simulink model "Simulink_Program"  (R2023b)
settings: Solver=FixedStepAuto  StartTime=0.0  StopTime=20  FixedStep=0.001  RelTol=1e-3
PreLoadFcn:
  | % NXP_MBDT_S32K3 callback: DO NOT REMOVE
  | mbd_s32k3.common.nxp.model_callbacks.preLoadFcn();
PostLoadFcn:
  | % NXP_MBDT_S32K3 callback: DO NOT REMOVE
  | mbd_s32k3.common.nxp.model_callbacks.postLoadFcn();
InitFcn:
  | % NXP_MBDT_S32K3 callback: DO NOT REMOVE
  | mbd_s32k3.common.nxp.model_callbacks.initFcn();
undefined symbols (not defined in the file or in its folder): battery_builder_rtmsupport, DiscIntNeverNeededParam, gcbh
libraries: nesl_utility x116, pack_105s34p_structured_with_balancing_lib x105, fl_lib x16, ee_lib x7, simulink x5, BatteryBalancing x1
(part 8 of 8)

== matlab function Simulink_Program/Subsystem/  /contactorControl ==
  settings: updateMethod=DISCRETE
  data: Fault_HVBatRdy input; VCU_DriveReady input; VCU_MainHighVltCntctrCmd input; VCU_DC_CntctrCmd input; Sensors_HVBatteryCurrent_BEV input; Sensors_HVBatteryVoltage_BEV input; Sensors_HVBatteryVoltage_BEV_DCLink input; Sensors_HVBatteryVoltage_BEV_DCFC input; Sensors_HVBatPrecharge_Temp input; Rprecharge input; HVIL_HVBatIntrlk_InternalStat input; K1 output; HVBatPrechgInbt input; K2 output; K3 output; K4 output; HVBatCntctrStat output; Ke_t_HighVoltageBusActualPrechargeTime output; Ke_I_HighVoltageBusShortageCurrent output; Ke_V_HighVoltagePreChargeThreshold output; HVBatPrechgInbt output
function [K1, K2, K3, K4, HVBatCntctrStat, Ke_t_HighVoltageBusActualPrechargeTime, Ke_I_HighVoltageBusShortageCurrent, Ke_V_HighVoltagePreChargeThreshold, HVBatPrechgInbt] = fcn(Fault_HVBatRdy, VCU_DriveReady, VCU_MainHighVltCntctrCmd, VCU_DC_CntctrCmd, Sensors_HVBatteryCurrent_BEV, Sensors_HVBatteryVoltage_BEV, Sensors_HVBatteryVoltage_BEV_DCLink, Sensors_HVBatteryVoltage_BEV_DCFC, Sensors_HVBatPrecharge_Temp, Rprecharge, HVIL_HVBatIntrlk_InternalStat, HVBatPrechgInbt)
%-----------------------------------------------------------
% McMaster Automotive Resource Centre (MARC)
% Electrical Engineer: Romulo Vieira, M.Sc and MBA - PhD Candidate
%-----------------------------------------------------------
% Purpose: Manage precharge relay and contactor sequence with retry + inhibit logic
%-----------------------------------------------------------
% HVBatCntctrStat Mapping:
% 0 – Idle/Waiting
% 1 – Precharge Active
% 2 – Precharge Complete (Main contactors closed)
% 3 – Precharge Failed (necessary cooldown after unique failures of voltage and current)
% 4 – Inhibit State (necessary cooldown after multiple failures)
% 5 - DCFC Charging
% 6 – Contactor Control Shorted High
% 7 - Contactor Control Shorted Low
% VCU_MainHighVltCntctrCmd mapping:
% 0 - open
% 1 - closed
% 2 - impact_open
% 3 - fast_open
% 7 - invalid
% --- Constants ---
Unset_VbusTargetHitIdx             = -1;   % Flag indicating Vbus threshold not yet hit
Min_Samples                        = 0;    % Minimum number of samples where relays and contactor are both active
% Internal Configuration Parameters
Ke_t_PrechargeFailCoolTime             = 1000;   % Cooldown time after one single failed attempt [ms]
Ke_t_ExtraPrechargeFailCoolPenaltyTime = 1000;   % Additional cooldown after two multiple failures [ms]
Ke_t_PrechargeFailMaxPenalty           = 10000;  % Maximum time threshold before enabling precharge inhibit state
Ke_t_BPCM_Precharge_Fail_Penalty_Time  = 15000;  % Hard inhibit time if failures persist [ms]
Ke_I_HighVoltageBusShortageCurrentMin  = 10.8;   % Minimum expected precharge current [A] (Pack current ≥ 0.6 * (pack voltage / Precharge resistance))
                                                 % // max pack voltage = 450 V, Precharge resistance = 25 ohm
Ke_V_HighVoltagePreChargeThreshold     = 0.98 * Sensors_HVBatteryVoltage_BEV;  % Target voltage threshold
Ke_t_HighVoltageBusPrechargeTime       = 100;    % Maximum allowable time for precharging [ms]
% --- Persistent Variables ---
persistent I_buffer idx HVBatCntctrStat_Aux VbusTargetHitIdx ...
    t_counter last_PrechargeAllw Prchrgpnltytimer PrechargeFailCount last_HVBatCntctrStat_Aux
if isempty(I_buffer)
    I_buffer               = zeros(Ke_t_HighVoltageBusPrechargeTime,1);
    idx                    = 1;
    HVBatCntctrStat_Aux    = 0;
    VbusTargetHitIdx       = Unset_VbusTargetHitIdx;
    t_counter              = 0;
    last_PrechargeAllw     = 0;
    Prchrgpnltytimer       = 0;
    PrechargeFailCount     = 0; % Represented by k_fail in the flowchart
    last_HVBatCntctrStat_Aux = 0;
end
% --- Default Outputs ---
K1 = 0; K2 = 0; K3 = 0; K4 = 0;
HVBatCntctrStat = HVBatCntctrStat_Aux;
Ke_t_HighVoltageBusActualPrechargeTime = 0;
Ke_I_HighVoltageBusShortageCurrent = 0;
if VCU_MainHighVltCntctrCmd == 3 % VCU_MainHighVltCntctrCmd = 3 means FAST_OPEN
    HVBatCntctrStat_Aux = 0;
end
% --- State Machine ---
switch HVBatCntctrStat_Aux
    case 0 % OPEN STATE
        % Set the Battery Contactor States
        K1 = 0; K2 = 0; K3 = 0; K4 = 0;
        % Check Initial conditions
        % HVIL_HVBatIntrlk_InternalStat = 1 (pass),
        % HVIL_HVBatIntrlk_InternalStat = 2 (fail)
        % For precharge initiation HVIL_HVBatIntrlk_InternalStat must pass.
        if VCU_MainHighVltCntctrCmd == 1 && HVBatPrechgInbt == 0 && HVIL_HVBatIntrlk_InternalStat == 1 && Fault_HVBatRdy == 1 && VCU_DriveReady == 1 && Sensors_HVBatPrecharge_Temp < 60
            I_buffer(:) = 0;
            idx = 1;
            VbusTargetHitIdx = Unset_VbusTargetHitIdx;
            t_counter = 0;
            HVBatCntctrStat_Aux = 1;
        end

        %% Shorted High
        
        if VCU_MainHighVltCntctrCmd == 0 && Sensors_HVBatteryVoltage_BEV_DCLink > 270 
            HVBatCntctrStat_Aux = 6;
        end

    case 1  % PRECHARGING STATE
        % Abort if initial conditions are no longer valid
        if VCU_MainHighVltCntctrCmd == 0 || HVBatPrechgInbt == 1 || HVIL_HVBatIntrlk_InternalStat == 2 || Fault_HVBatRdy == 0 || VCU_DriveReady == 0 || Sensors_HVBatPrecharge_Temp >= 60
            HVBatCntctrStat_Aux = 0;
        end
        % Set precharge relays ON
        K1 = 0; K2 = 1; K3 = 1; K4 = 0;
        %
        % if Fault_K1_Shorted_Low_Condition == 1
        %     HVBatCntctrStat_Aux = 7; % K1 Shorted Low
        % end
        % if Fault_K4_Shorted_Low_Condition == 1
        %     HVBatCntctrStat_Aux = 7; % K4 Shorted Low
        % end
        % Record current
        if t_counter == 0
            I_buffer(idx) = Sensors_HVBatteryVoltage_BEV / Rprecharge;
        else
            I_buffer(idx) = Sensors_HVBatteryCurrent_BEV;
        end
        Ke_I_HighVoltageBusShortageCurrent = max(I_buffer);
        % Voltage target check
        if VbusTargetHitIdx == Unset_VbusTargetHitIdx && Sensors_HVBatteryVoltage_BEV_DCLink >= Ke_V_HighVoltagePreChargeThreshold
            VbusTargetHitIdx = t_counter + 1;
        end
        % Time since start or since target hit
        if VbusTargetHitIdx ~= Unset_VbusTargetHitIdx
            delta_samples = VbusTargetHitIdx;
        else
            delta_samples = t_counter;
        end
        % SUCCESS - PRECHARGE CONDITIONS ARE MET
        if t_counter < Ke_t_HighVoltageBusPrechargeTime && ...
                VbusTargetHitIdx ~= Unset_VbusTargetHitIdx && delta_samples < Ke_t_HighVoltageBusPrechargeTime && Ke_I_HighVoltageBusShortageCurrent >= Ke_I_HighVoltageBusShortageCurrentMin
            
            % Set the next Battery Contactor Status
            HVBatCntctrStat_Aux = 2;
            % Set the Battery Contactor States
            K1 = 1; K2 = 0; K3 = 0; K4 = 0;
            PrechargeFailCount = 0;
            Prchrgpnltytimer = 0;
        % FAILURE - PRECHARGE CONDITIONS ARE NOT MET
        elseif t_counter == Ke_t_HighVoltageBusPrechargeTime && ...
               (VbusTargetHitIdx == Unset_VbusTargetHitIdx || delta_samples == Ke_t_HighVoltageBusPrechargeTime || Ke_I_HighVoltageBusShortageCurrent < Ke_I_HighVoltageBusShortageCurrentMin)
            % Set the Battery Contactor States
            K1 = 0; K2 = 0; K3 = 0; K4 = 0;
           % Set the Battery Contactor Status
            HVBatCntctrStat_Aux = 3;
            % Update the total cooling time before re-attempt
            PrechargeFailCount = PrechargeFailCount + 1;
            Prchrgpnltytimer = Prchrgpnltytimer + Ke_t_PrechargeFailCoolTime;
            if PrechargeFailCount >= 2
                Prchrgpnltytimer = Ke_t_ExtraPrechargeFailCoolPenaltyTime;
            end
            if PrechargeFailCount >= 10
                HVBatCntctrStat_Aux = 4;
                HVBatPrechgInbt = 1;
                Prchrgpnltytimer = Ke_t_BPCM_Precharge_Fail_Penalty_Time;
            end
        end
        % Update counters
        t_counter = t_counter + 1;
        idx = idx + 1;
        if idx > Ke_t_HighVoltageBusPrechargeTime, idx = 1; end
    case 2  % PRECHARGE COMPLETE OR CLOSED
        % Set the Battery Contactor States
        K1 = 1; K2 = 0; K3 = 0; K4 = 0;
        % If statement to detect DCFC charging
        if VCU_DC_CntctrCmd == 1
            HVBatCntctrStat_Aux = 5; % DCFC CHARGING
        end
        if VCU_MainHighVltCntctrCmd == 0
            HVBatCntctrStat_Aux = 0;
        end

        if VCU_MainHighVltCntctrCmd == 1 && Sensors_HVBatteryVoltage_BEV_DCLink < 270
            HVBatCntctrStat_Aux = 7;
        end

    case 3 % FAULT: PRECHARGE FAILED STATE 4 --> 3
        % Set the Battery Contactor States
        K1 = 0; K2 = 0; K3 = 0; K4 = 0;
        if Prchrgpnltytimer > 0
            Prchrgpnltytimer = Prchrgpnltytimer - 1;
        else
            HVBatCntctrStat_Aux = 0;
        end
    case 4  % FAULT: PRECHARGE INHIBIT STATE - 5 -- > 4
        % Set the Battery Contactor States
        K1 = 0; K2 = 0; K3 = 0; K4 = 0;
        if Prchrgpnltytimer > 0
            Prchrgpnltytimer = Prchrgpnltytimer - 1;
        else
        Prchrgpnltytimer = 0;
        HVBatPrechgInbt = 0;
        HVBatCntctrStat_Aux = 0;
        PrechargeFailCount = 0;
        end
    % Added by Tridib: New DCFC case -3 -- > 5
    case 5 % DCFC CHARGING
        % Set the Battery Contactor States
        K1 = 1; K2 = 0; K3 = 0; K4 = 1;
        if VCU_DC_CntctrCmd == 0
            if VCU_MainHighVltCntctrCmd == 0
                HVBatCntctrStat_Aux = 0; % switch to OPEN
            else
                HVBatCntctrStat_Aux = 2; % switch to CLOSED
            end
        end
    
    case 6 % FAULT: Shorted High
       
        if VCU_MainHighVltCntctrCmd == 0 && Sensors_HVBatteryVoltage_BEV_DCLink < 270
            HVBatCntctrStat_Aux = 0;
        end

    case 7 % FAULT: Shorted Low

        if VCU_DC_CntctrCmd == 0 && Sensors_HVBatteryVoltage_BEV_DCFC < 270 && VCU_MainHighVltCntctrCmd == 1 && Sensors_HVBatteryVoltage_BEV_DCLink > 270
            HVBatCntctrStat_Aux = 2;
        end
    otherwise
        K1 = 0; K2 = 0; K3 = 0; K4 = 0;
        HVBatCntctrStat_Aux = 0;
end
% --- Final Outputs ---
HVBatCntctrStat = HVBatCntctrStat_Aux;
Ke_t_HighVoltageBusActualPrechargeTime = max(0, VbusTargetHitIdx + ...
    Min_Samples * (VbusTargetHitIdx ~= Unset_VbusTargetHitIdx));
last_PrechargeAllw = double(Fault_HVBatRdy);
last_HVBatCntctrStat_Aux = HVBatCntctrStat_Aux;
end

== matlab function Simulink_Program/Subsystem/Cell Balancing/MATLAB Function ==
  data: HVBatCell_Voltages input; BalCmd output; Enable input; isBalancingActive output

function [BalCmd, isBalancingActive] = PassiveBalanceController(HVBatCell_Voltages, Enable)
% PassiveBalanceController - Controls passive cell balancing and detects faults
% Inputs:
%   HVBatCell_Voltages - Vector of cell voltages
%   Enable - Boolean flag to enable balancing
% Outputs:
%   BalCmd - Logical vector of balancing commands (true = balance)
%   isBalancingActive - Logical flag indicating if any balancing is active

%#codegen

% Parameters
V_thresh = 0.05; % Threshold in Volts (50 mV)
N = length(HVBatCell_Voltages);
BalCmd = false(N, 1);

% Check if balancing is enabled
if Enable
    % Find minimum cell voltage
    V_min = min(HVBatCell_Voltages);

    % Loop through each cell
    for i = 1:N
        deltaV = HVBatCell_Voltages(i) - V_min;

        % Normal balancing condition
        if deltaV > V_thresh
            BalCmd(i) = true;
        else
            BalCmd(i) = false;
        end

        % Fault detection (optional logging)
        if deltaV <= V_thresh && BalCmd(i)
            fprintf('Stuck Closed Fault detected on Cell %d\n', i);
        elseif deltaV > V_thresh && ~BalCmd(i)
            fprintf('Stuck Open Fault detected on Cell %d\n', i);
        end
    end
end

% Balancing active flag: at least one command is true
isBalancingActive = any(BalCmd);

end

== matlab function Simulink_Program/Subsystem/Plug-In Charging/ac_plugin_charging ==
  settings: updateMethod=DISCRETE
  data: HVBat_DC_CntctrStat output; Time input; DCChargeInitialized input; MainHighVltCntctrCmd input; ChargingSysSts input; Charger_Plugin_Status input; HVBatCell_Voltage_High_Thrsh input; Fault_General input; HVBatModTempMax input; HVBatCellVltMax input; HVBatSOCMax input; K1 input; K2 input; K3 input; HVBatChargeStat output; MaxChargeCurrentAllowed output; MaxChargeRegenPowerAllowed output; HVBatMaxCellVltAlld output; HVBatMaxPkVltAllwd output
function [HVBat_DC_CntctrStat, HVBatChargeStat, MaxChargeCurrentAllowed, MaxChargeRegenPowerAllowed, HVBatMaxCellVltAlld, HVBatMaxPkVltAllwd] = ac( ...
    Time, DCChargeInitialized, MainHighVltCntctrCmd, ChargingSysSts, Charger_Plugin_Status, ...
    HVBatCell_Voltage_High_Thrsh, Fault_General, ...
    HVBatModTempMax, HVBatCellVltMax, HVBatSOCMax, K1, K2, K3)

% ===========================
%% INPUTS
% Time - Current time (s)
% DC_CntctrCmd - Command from VCU to close DCFC contactor (0/1/2/3/7)
% MainHighVltCntctrCmd - VCU command for main contactor (0/1/2/3/7)
% K4,K3,K2,K1 - Physical contactor feedbacks (1=closed, 0=open)
% Fault_General - Fault flag (0=OK, 1=Fault/Weld)
% ChargingSysSts - 0=Not Charging,1=Charging,2=Interrupted,3=Complete
% Charger_Plugin_Status - 0=Unplugged, 1=Plugged
% HVBatCell_Voltage_High_Thrsh - Max allowable cell voltage (V)
% HVBatModTempMax, HVBatCellVltMax, HVBatSOCMax - vectors
% VDC - DC bus voltage (V)
% HVBatMaxPkVltAllwd 
% HVBatMaxCellVltAlld

%% OUTPUTS
% HVBat_DC_CntctrStat - 0=Open, 1=Closed, 2=Faulted
% HVBatChargeStat     - 0=Not Ready, 1=Ready, 2=Charging, 3=Fault, 6=Complete
% MaxChargeCurrentAllowed - Allowed charging current (A)
% P_charge            - Estimated DC charging power (W)

%% --- Lookup Table for Max Charge Current [SOC vs Temp] ---
SOC = [0 0.05 0.10 0.15 0.20 0.25 0.30 0.35 0.40 0.45 0.50 0.55 0.60 0.65 0.70 0.75 0.80 0.85 0.90 0.95 1.00]; % 19 + 2 points
Temp = [-25 -20 -10 0 5 10 20 25 35 40 50]; % 11 points

ACChgCurrentProfile = [ ...
  0.05 0.05 0.05 0.05 0.05 0.05 0.05 0.05 0.05 0.05 0.05 0.05 0.05 0.02 0.02 0.01 0.01 0.01 0.01 0.01 0.00; ...
  0.06 0.06 0.06 0.06 0.06 0.06 0.06 0.06 0.06 0.06 0.06 0.06 0.06 0.02 0.02 0.02 0.02 0.01 0.01 0.01 0.00; ...
  0.45 0.45 0.45 0.45 0.45 0.15 0.15 0.15 0.15 0.09 0.09 0.09 0.09 0.03 0.03 0.03 0.03 0.02 0.02 0.02 0.00; ...
  0.70 0.70 0.70 0.70 0.70 0.70 0.70 0.70 0.70 0.50 0.50 0.50 0.50 0.25 0.25 0.25 0.25 0.10 0.10 0.09 0.00; ...
  0.85 0.85 0.85 0.85 0.85 0.85 0.85 0.85 0.85 0.75 0.75 0.75 0.75 0.63 0.63 0.58 0.44 0.35 0.24 0.12 0.00; ...
  0.88 0.88 0.88 0.88 0.88 0.88 0.88 0.88 0.88 0.88 0.88 0.88 0.88 0.88 0.88 0.68 0.59 0.35 0.24 0.12 0.00; ...
  0.88 0.88 0.88 0.88 0.88 0.88 0.88 0.88 0.88 0.88 0.88 0.88 0.88 0.88 0.88 0.88 0.74 0.59 0.44 0.29 0.00; ...
  0.88 0.88 0.88 0.88 0.88 0.88 0.88 0.88 0.88 0.88 0.88 0.88 0.88 0.88 0.88 0.88 0.88 0.74 0.59 0.44 0.00; ...
  0.88 0.88 0.88 0.88 0.88 0.88 0.88 0.88 0.88 0.88 0.88 0.88 0.88 0.88 0.88 0.88 0.88 0.74 0.65 0.59 0.00; ...
  0.88 0.88 0.88 0.88 0.88 0.88 0.88 0.88 0.88 0.88 0.88 0.88 0.88 0.88 0.88 0.88 0.88 0.76 0.74 0.60 0.00; ...
  0.88 0.88 0.88 0.88 0.88 0.88 0.88 0.88 0.88 0.88 0.88 0.88 0.88 0.88 0.88 0.88 0.88 0.60 0.60 0.48 0.00 ...
];

%% --- Calibrations ---
persistent Ke_t_DCFC_CntctrOpn Ke_T_HighChrgTempThresholdIn Ke_T_LowChrgTempThresholdIn ...
           Ke_T_HighChrgTempThresholdOut Ke_T_LowChrgTempThresholdOut Ke_U_ChargeCompletionVlt ...
           ParallelCellNum Ke_t_ChargeCompletionHold HVBatChargeTimer ...
           chargeCompleteFlag overTempFlag
if isempty(Ke_t_DCFC_CntctrOpn)
    Ke_t_DCFC_CntctrOpn = 1.5;           
    Ke_T_HighChrgTempThresholdIn = 50;   
    Ke_T_LowChrgTempThresholdIn  = -25;  
    Ke_T_HighChrgTempThresholdOut = 48;  
    Ke_T_LowChrgTempThresholdOut  = -23; 
    Ke_U_ChargeCompletionVlt = 4.14;     
    ParallelCellNum = 34;
    Ke_t_ChargeCompletionHold = 0.3;     % seconds
    HVBatChargeTimer = 0;
    chargeCompleteFlag = false;
    overTempFlag = false;
end


persistent Ke_RegenVltMargin Ke_P_MaxChargeRegen
if isempty(Ke_RegenVltMargin)
    Ke_RegenVltMargin   = 0.05;   % [V] margin below HVBatCell_Voltage_High_Thrsh
    Ke_P_MaxChargeRegen = 11000;  % [W] max allowed regen during charge
end

%% --- Persistent states ---
persistent HVBatChargeStat_Aux DC_FaultDetectedTime DC_WeldDetected MaxChargeCurrentAllowed_Aux MaxChargeRegenPowerAllowed_Aux
if isempty(HVBatChargeStat_Aux)
    HVBatChargeStat_Aux = 0;
    DC_FaultDetectedTime = -1;
    DC_WeldDetected = false;
    MaxChargeCurrentAllowed_Aux = 0;
    MaxChargeRegenPowerAllowed_Aux = 0;
end

%% Default outputs
HVBatChargeStat = HVBatChargeStat_Aux;
MaxChargeCurrentAllowed = MaxChargeCurrentAllowed_Aux;
MaxChargeRegenPowerAllowed = MaxChargeRegenPowerAllowed_Aux;

%% Plug-in readiness
if (Charger_Plugin_Status == 1) && (DCChargeInitialized == 0) && ~chargeCompleteFlag && overTempFlag == false
    HVBatChargeStat_Aux = 0; % Not Ready
    % Battery is able to accept charge
    if (MainHighVltCntctrCmd == 1) && (K1 == 1) && (K2 == 0) && (K3 == 0) && ~chargeCompleteFlag && overTempFlag == false && Fault_General == 0
        HVBatChargeStat_Aux = 1;   % Ready
    elseif (MainHighVltCntctrCmd == 1) && (K1 == 1) && (K2 == 0) && (K3 == 0) && ~chargeCompleteFlag && overTempFlag == false && Fault_General == 1
        HVBatChargeStat_Aux = 0;   % Not Ready
    end
elseif Charger_Plugin_Status == 1 && DCChargeInitialized == 0 && chargeCompleteFlag && overTempFlag == false
        MaxChargeCurrentAllowed_Aux = 0;
        HVBatChargeStat_Aux = 6;       % Complete
else
    HVBatChargeStat_Aux = 0;
    MaxChargeCurrentAllowed_Aux = 0;
    MaxChargeRegenPowerAllowed_Aux = 0;
    DC_FaultDetectedTime = -1;
    DC_WeldDetected = false;
end

%% Charging logic
handshake_ok = (HVBatChargeStat_Aux == 1 && ChargingSysSts == 1);
if handshake_ok
    HVBatChargeStat_Aux = 2; % charge allow current
end

Vmax = max(HVBatCellVltMax);
Temp_actual = max(HVBatModTempMax);
SOC_actual  = max(HVBatSOCMax);

if overTempFlag == true
    if Temp_actual <= (Ke_T_HighChrgTempThresholdIn - 5) && Temp_actual >= (Ke_T_LowChrgTempThresholdIn + 5)
        overTempFlag = false;
    end
end

charge_allow_threshold = Ke_U_ChargeCompletionVlt;

if HVBatChargeStat_Aux == 2 && (ChargingSysSts == 1) && (Vmax < charge_allow_threshold) && chargeCompleteFlag == false && overTempFlag == false && Temp_actual < Ke_T_HighChrgTempThresholdIn && Temp_actual > Ke_T_LowChrgTempThresholdIn
    MaxChargeCurrentAllowed = ParallelCellNum * interp2(SOC, Temp, ACChgCurrentProfile, SOC_actual, Temp_actual);
      
    MaxChargeCurrentAllowed_Aux = MaxChargeCurrentAllowed;
    HVBatChargeStat_Aux = 2; % charge allow current
    
% Charge completion    
elseif (ChargingSysSts == 1) && (Vmax >= (HVBatCell_Voltage_High_Thrsh - 0.01))
        % Enter CV-hold mode: provide PackCVCurrent to hold voltage steady
        MaxChargeCurrentAllowed_Aux = 0;
        HVBatChargeStat_Aux = 6;       % Complete
        chargeCompleteFlag = true;

elseif Temp_actual >= Ke_T_HighChrgTempThresholdIn || Temp_actual <= Ke_T_LowChrgTempThresholdIn
    % Not in charging handshake/phase: ensure DCFC current 0
    MaxChargeCurrentAllowed_Aux = 0;
    overTempFlag = true;
    HVBatChargeStat_Aux = 3;
end

if ChargingSysSts == 1
    %% Regen power limit during charge mode
     Vcell_max = max(HVBatCellVltMax);

    if Vcell_max >= HVBatCell_Voltage_High_Thrsh
        % No regen allowed at or above max allowable cell voltage
        MaxChargeRegenPowerAllowed = 0;
        MaxChargeRegenPowerAllowed_Aux = MaxChargeRegenPowerAllowed;

    elseif Vcell_max >= (HVBatCell_Voltage_High_Thrsh - Ke_RegenVltMargin)
        % Linearly reduce regen as voltage approaches limit
        scale = (HVBatCell_Voltage_High_Thrsh - Vcell_max) / Ke_RegenVltMargin;
        MaxChargeRegenPowerAllowed = max(0, scale * Ke_P_MaxChargeRegen);
        MaxChargeRegenPowerAllowed_Aux = MaxChargeRegenPowerAllowed;

    else
        % Full regen allowed (charge-mode limited)
        MaxChargeRegenPowerAllowed = Ke_P_MaxChargeRegen;
        MaxChargeRegenPowerAllowed_Aux = MaxChargeRegenPowerAllowed;
    end
end

% Fault handling
if ChargingSysSts == 1 && Fault_General == 1 
    HVBatChargeStat_Aux = 3;
    %P_charge = 0;
else
    DC_FaultDetectedTime = -1;
end

%% Charge completion
if max(HVBatCellVltMax) >= Ke_U_ChargeCompletionVlt
    % if HVBatChargeTimer < 0
    %     HVBatChargeTimer = Time; % latch the first detection time
    % end
    % 
    % % Check if hold duration has passed
    % if (Time - HVBatChargeTimer) >= Ke_t_ChargeCompletionHold
        MaxChargeCurrentAllowed_Aux = 0;
        HVBatChargeStat_Aux = 6;
        chargeCompleteFlag = true;
        MaxChargeRegenPowerAllowed_Aux = 0;
    % end
else
    HVBatChargeTimer = -1; % reset latch when voltage drops below threshold
    chargeCompleteFlag = false;
end

if (ChargingSysSts == 3)
    MaxChargeCurrentAllowed_Aux = 0;
    HVBatChargeStat_Aux = 6;       % Complete
    chargeCompleteFlag = true;
end

%% Overvoltage handling
if max(HVBatCellVltMax) > HVBatCell_Voltage_High_Thrsh
    HVBatChargeStat_Aux = 3;
    % P_charge = 0;
end

%% Outputs
HVBatChargeStat = HVBatChargeStat_Aux;
MaxChargeCurrentAllowed = MaxChargeCurrentAllowed_Aux;
MaxChargeRegenPowerAllowed = MaxChargeRegenPowerAllowed_Aux;
if isnan(MaxChargeCurrentAllowed), MaxChargeCurrentAllowed = 0; end
if isnan(MaxChargeRegenPowerAllowed), MaxChargeRegenPowerAllowed = 0; end

%% --- Step 3: Update Max Voltages
HVBatMaxCellVltAlld = HVBatCell_Voltage_High_Thrsh;
HVBatMaxPkVltAllwd = HVBatCell_Voltage_High_Thrsh * 105; % example for 105 series cells

HVBat_DC_CntctrStat = 0;
end

== matlab function Simulink_Program/Subsystem/Plug-In Charging/MATLAB Function ==
  data: DCChargeInitialized input; MaxChargeCurrentAllowed_AC input; output output; MaxChargeCurrentAllowed_DC input
function output = fcn(DCChargeInitialized, MaxChargeCurrentAllowed_AC, MaxChargeCurrentAllowed_DC)
    if DCChargeInitialized == 0
        output = MaxChargeCurrentAllowed_AC;
    else
        output = MaxChargeCurrentAllowed_DC;
    end

== matlab function Simulink_Program/Subsystem/Plug-In Charging/dcfc ==
  settings: updateMethod=DISCRETE
  data: DC_Isolation_Sts output; HVBat_DC_CntctrStat output; HVBatChargeStat output; Time input; DCChargeInitialized input; MaxChargeCurrentAllowed output; PlugInChargingCmd input; DC_Isolation_Cmd input; MainHVBatCntctrCmd input; ChargingSysSts input; Charger_Plugin_Status input; HVBatCell_Voltage_High_Thrsh input; Fault_General input; temperatureCell input; voltageCell input; socCell input; K1 input; K2 input; K3 input; HVBat_DC_CntctrOpn output; HVBatCntctrOpn output; HVBatCntctrReq output; K4 input
function [DC_Isolation_Sts, HVBat_DC_CntctrStat, HVBatChargeStat, MaxChargeCurrentAllowed, HVBat_DC_CntctrOpn, HVBatCntctrOpn, HVBatCntctrReq] = dcfc(Time, DCChargeInitialized, PlugInChargingCmd, DC_Isolation_Cmd, MainHVBatCntctrCmd, ChargingSysSts, Charger_Plugin_Status, HVBatCell_Voltage_High_Thrsh, Fault_General, temperatureCell, voltageCell, socCell, K1, K2, K3, K4)

% ===========================
%% INPUTS
% Time - current absolute time (s)
% PlugInChargingCmd - Command from VCU indicating vehicle is plugged into DCFC station and ready to close DCFC contactor (K4) (0 - Open, 1 - Closed, 2 - Impact Open, 3 - Fast Open, 7 - SNA).
% MainHVBatCntctrCmd - Command from VCU indicating main contactor (K1) is commanded to close (0 - Open, 1 - Closed, 2 - Impact Open, 3 - Fast Open, 7 - SNA).
% K4 - Physical feedback (status of DCFC contactor) (1 - physically closed, 0 - physically open).
% K3 - Physical feedback (status of precharge (+) contactor) (1 - physically closed, 0 - physically open).
% K2 - Physical feedback (status of precharge (-) contactor) (1 - physically closed, 0 - physically open).
% K1 - Physical feedback (status of main contactor) (1 - physically closed, 0 - physically open).
% Fault_General - Indicate if DCFC contactor driver circuit fault or a weld is detected (0 - normal, 1 - at least 1 fault detected).
% DC_Isolation_Cmd - Command from VCU to disable isolation monitoring when DCFC active (0 - Enable, 1 - Disable).
% ChargingSysSts - Command from VCU and indicates the status of the charging function (0 - Not Charging, 1 - Charging, 2 - ChargeInterrupted, 3 - ChargeComplete, 7 - SNA). 
% Charger_Plugin_Status - Signal from charge port. True if DCFC connector is plugged in (0 - Charger_Unplugged, 1 - Charger_Plugged_In).
% HVBatPack_Voltage - Current HV pack voltage (V).
% MaxCellVoltage_V - Highest measured cell voltage (V).
% HVBatCell_Voltage_High_Thrsh - Signal from the BPCM indicating the maximum cell voltage that the battery pack needs to be below in order to become functional. Above this value, the BPCM will set HVBatCntctrReq to 1, and HVBatCntctrOpn to 1 after appropriate maturation time. If above this value during power up, HVBatRdy will also be set to 0.
% cellTemperature - All cell temperatures in degC.
% cellVoltage - All cell voltages in V.
% cellSOC - All cell SOCs in percentages.
% ===========================
%% OUTPUTS
% HVBat_DC_CntctrStat - DCFC contactor status (0 - Open, 1 - Closed, 2 - Faulted).
% HVBatChargeStat - Sent out by the BPCM as an indication of its present status (0 - Not Ready, 1 - Ready, 2 - Complete).
% DC_Isolation_Sts - Isolation status for DC charging (0 - Enable, 1 - Disable).
% HVBatMaxCellVltAlld - Max allowable cell voltage (V).
% HVBatMaxPkVltAllwd - Max allowable pack voltage (V).
% MaxChargeCurrentAllowed - Max allowable DCFC current (A).
% HVBatCntctrReq - Request to open contactors (fault/weld) (0 - No request to open, 1 - Request to open).
% HVBatCntctrOpn - Command to DCFC contactor coil (Not Commanded Open, 1 - Commanded Open).
%% CALIBRATIONS (Constants/Tunable Parameters)
% MaxChargeCurrentDefault - Max allowable DCFC charging current (Default = 30 A).
% Ke_T_HighChrgTempThresholdIn - Upper limit for enabling charging (Default = 50 deg C).
% Ke_T_HighChrgTempThresholdOut - Upper hysteresis threshold (Default = 48 deg C).
% Ke_T_LowChrgTempThresholdIn - Lower limit for enabling charging (Default = -25 deg C).
% Ke_T_LowChrgTempThresholdOut - Lower hysteresis threshold (Default = -23 deg C).
% Ke_U_ChargeCompletionVlt - Voltage threshold for charge completion (Default = 4.14 V).
% Ke_t_DCFC_CntctrOpn - Delay before opening contactor after fault (Default = 1.5 sec).
% ParallelCellNum - Number of parallel cell, which dictates pack current (34).
%% --- Lookup Table for AC Max Charge Current [SOC vs Temp]
SOC = [0 0.05 0.10 0.15 0.20 0.25 0.30 0.35 0.40 0.45 0.50 0.55 0.60 0.65 0.70 0.75 0.80 0.85 0.90 0.95 1.00]; % 19 + 2 points
Temp = [-25 -20 -10 0 5 10 20 25 35 40 50]; % 11 points
DCChgCurrentProfile = [ ...
  0.05 0.05 0.05 0.05 0.05 0.05 0.05 0.05 0.05 0.05 0.05 0.05 0.05 0.02 0.02 0.01 0.01 0.01 0.01 0.01 0.00; ...
  0.06 0.06 0.06 0.06 0.06 0.06 0.06 0.06 0.06 0.06 0.06 0.06 0.06 0.02 0.02 0.02 0.02 0.01 0.01 0.01 0.00; ...
  0.45 0.45 0.45 0.45 0.45 0.15 0.15 0.15 0.15 0.09 0.09 0.09 0.09 0.03 0.03 0.03 0.03 0.02 0.02 0.02 0.00; ...
  0.70 0.70 0.70 0.70 0.70 0.70 0.70 0.70 0.70 0.50 0.50 0.50 0.50 0.25 0.25 0.25 0.25 0.10 0.10 0.10 0.00; ...
  0.85 0.85 0.85 0.85 0.85 0.85 0.85 0.85 0.85 0.75 0.75 0.75 0.75 0.63 0.63 0.58 0.58 0.35 0.35 0.20 0.00; ...
  1.00 1.00 1.00 1.00 1.00 1.00 1.00 1.00 1.00 1.00 1.00 1.00 1.00 1.00 1.00 0.90 0.90 0.60 0.60 0.30 0.00; ...
  1.33 1.33 1.33 1.33 1.33 1.33 1.33 1.33 1.33 1.33 1.33 1.33 1.33 1.33 1.33 1.30 1.30 0.80 0.80 0.50 0.00; ...
  1.50 1.50 1.50 1.50 1.50 1.50 1.50 1.50 1.50 1.50 1.50 1.50 1.50 1.50 1.50 1.50 1.50 0.90 0.90 0.60 0.00; ...
  2.17 2.17 2.17 2.17 2.17 2.17 2.17 2.17 2.17 2.17 2.17 2.17 2.17 1.83 1.83 1.50 1.50 0.90 0.90 0.60 0.00; ...
  2.50 2.50 2.50 2.50 2.50 2.50 2.50 2.50 2.50 2.50 2.50 2.50 2.50 2.00 2.00 1.50 1.50 0.90 0.90 0.60 0.00; ...
  1.50 1.50 1.50 1.50 1.50 1.50 1.50 1.50 1.50 1.50 1.50 1.50 1.50 1.50 1.50 1.00 1.00 0.60 0.60 0.48 0.00  ...
];
%% --- Calibration (tunable) ---
% --- Contactor timing calibrations ---
persistent MaxChargeCurrentDefault ...
           Ke_T_HighChrgTempThresholdIn Ke_T_LowChrgTempThresholdIn ...
           Ke_T_HighChrgTempThresholdOut Ke_T_LowChrgTempThresholdOut ...
           Ke_U_ChargeCompletionVlt Ke_U_ChargeCompletionHyst ...
           ParallelCellNum Ke_t_ChargeCompletionHold  ...
           Ke_CV_PerCell_A Ke_MinCV_PackCurrent Ke_DisableBalDuringCV ...
           chargeCompleteFlag overTempFlag ...
           Ke_t_DCFC_CntctrOpn Ke_t_CntctrReqDelay
if isempty(MaxChargeCurrentDefault)
    % Ke_t_DCFC_CntctrOpn = 1.5;           % seconds (1500 ms) - time between HVBatCntctrOpn and actual coil open
    % Ke_t_CntctrReqDelay = 30.0;          % seconds - time HVBatCntctrReq must persist before notifying HVBatCntctrOpn
    Ke_t_DCFC_CntctrOpn = 0;
    Ke_t_CntctrReqDelay = 0;  
    MaxChargeCurrentDefault = 30;        % A (plugin default)
    Ke_T_HighChrgTempThresholdIn = 50;   % degC
    Ke_T_LowChrgTempThresholdIn  = -25;  % degC
    Ke_T_HighChrgTempThresholdOut = 48;  % degC
    Ke_T_LowChrgTempThresholdOut  = -23; % degC
    Ke_U_ChargeCompletionVlt = 4.19;     % V per cell held for completion (example)
    Ke_U_ChargeCompletionHyst = 0.005;   % 5 mV hysteresis (tunable)
    ParallelCellNum = 34; 
    Ke_t_ChargeCompletionHold = 0.3;     % seconds
    % CV-hold calibration (tunable)
    Ke_CV_PerCell_A = 0.06;              % A per cell for CV hold (eg 0.02C for 3Ah cell = 0.06A)
    Ke_MinCV_PackCurrent = 2.0;          % minimum pack-level CV current (A)
    Ke_DisableBalDuringCV = 1;           % 1 = attempt to disable passive balancing during CV-hold (set to 0 if unsupported)
    chargeCompleteFlag = false;
    overTempFlag = false;
end

%% --- Persistent runtime state ---
persistent HVBatChargeStat_Aux DC_Isolation_Sts_Aux HVBat_DC_CntctrStat_Aux MaxChargeCurrentAllowed_Aux DC_Cntctr_Opn_Aux HVBatChargeTimer
persistent HVBatCntctrReq_Aux HVBatCntctrOpn_Aux CntctrReqStartTime CntctrOpnStartTime
if isempty(HVBatChargeStat_Aux)
    HVBatChargeStat_Aux = 0;
    DC_Isolation_Sts_Aux = 0;
    HVBat_DC_CntctrStat_Aux = 0;
    MaxChargeCurrentAllowed_Aux = 0;
    HVBatChargeTimer = -1;
    DC_Cntctr_Opn_Aux = 0;

    % Contactor request/opn runtime state
    HVBatCntctrReq_Aux = 0;     % immediate request flag
    HVBatCntctrOpn_Aux = 0;     % "will open within Ke_t_DCFC_CntctrOpn" notification
    CntctrReqStartTime = -1;    % when request first asserted
    CntctrOpnStartTime = -1;    % when opn notification first asserted
end


%% Default outputs
HVBat_DC_CntctrStat = 0;
HVBatChargeStat = HVBatChargeStat_Aux;
DC_Isolation_Sts = DC_Isolation_Sts_Aux;
MaxChargeCurrentAllowed = MaxChargeCurrentAllowed_Aux;
HVBat_DC_CntctrOpn = DC_Cntctr_Opn_Aux;

% --- REMOVED Global Fault Detection Block ---

%% ---------- Step: Continuous isolation detection handling ----------
% Requirement: run continuous isolation detection whenever DC_Isolation_Cmd == 0
% and provide isolation status when DC_Isolation_Disable_Sts = ENABLE.
% Here we model DC_Isolation_Sts = DC_Isolation_Cmd (1=Disabled, 0=Enabled)    
if DC_Isolation_Cmd == 0
    DC_Isolation_Sts_Aux = 0; % ENABLED and continuous detection runs (model assumes OK)
elseif DC_Isolation_Cmd == 1
    DC_Isolation_Sts_Aux = 1; % DISABLED
end
DC_Isolation_Sts = DC_Isolation_Sts_Aux;

%% ---------- Step: Plug detection and readiness ----------
% This block now only handles non-fault states
if Charger_Plugin_Status == 1 && DCChargeInitialized == 1 && ~chargeCompleteFlag && overTempFlag == false
    % initial state: not ready until main contactor confirmed closed
    HVBatChargeStat_Aux = 0; % Not Ready
    % main contactor closure commanded by VCU (MainHVBatCntctrCmd) and confirmed via K1
    % precharge contactors K2,K3 should be open (0) at final close
    if MainHVBatCntctrCmd == 1 && K1 == 1 && K2 == 0 && K3 == 0 && ~chargeCompleteFlag && overTempFlag == false
        HVBatChargeStat_Aux = 1; % Ready
    end
elseif Charger_Plugin_Status == 1 && DCChargeInitialized == 1 && chargeCompleteFlag && overTempFlag == false
        MaxChargeCurrentAllowed_Aux = 0;
        HVBat_DC_CntctrStat_Aux = 0;   % Open
        HVBatChargeStat_Aux = 6;       % Complete
        DC_Isolation_Sts_Aux = 0;          % Enabled
else
    % unplugged: reset readiness and clear DC-specific states
    HVBatChargeStat_Aux = 0;
    HVBat_DC_CntctrStat_Aux = 0;
    MaxChargeCurrentAllowed_Aux = 0;
end
%% ---------- Step: DC contactor commanded by VCU and BPCM reporting ----------
% VCU commands DC contactor close via PlugInChargingCmd (this is DC_CntctrCmd renamed).
% When VCU commanded close and actual feedback K4 == 1, BPCM reports Closed.
% If commanded closed but K4 never closes and a fault is detected, BPCM reports Faulted.

% --- REFACTORED ---
% This block is now *only* responsible for non-fault contactor states.
% The global fault logic will override this if a fault is active.
if PlugInChargingCmd == 1 && chargeCompleteFlag == false && overTempFlag == false
    % VCU requested closure; check feedback
    if K4 == 1
        HVBat_DC_CntctrStat_Aux = 1; % Closed
    else
        % K4 not closed yet.
        HVBat_DC_CntctrStat_Aux = 0; % Open
    end
elseif PlugInChargingCmd == 1 && chargeCompleteFlag == true
    HVBat_DC_CntctrStat_Aux = 0; % Open
elseif PlugInChargingCmd == 1 && overTempFlag == true
    HVBat_DC_CntctrStat_Aux = 0; % Open
    HVBatChargeStat_Aux = 3; % Charge Disallow current
else
    % VCU is not commanding close => report open unless K4 is physically closed (safety anomaly)
    if K4 == 1
        HVBat_DC_CntctrStat_Aux = 1; % Closed (anomalous but reflect feedback)
    else
        HVBat_DC_CntctrStat_Aux = 0; % Open
    end
end
%% ---------- Step: Charging handshake and DCFC charging stage ----------
%  - DC_Isolation_Sts_Aux == 1 (DISABLE)  <-- requirement says disable before DCFC
%  - HVBat_DC_CntctrStat_Aux == 1 (Closed)
%  - ChargingSysSts == 1 (Charging)
handshake_ok = (HVBatChargeStat_Aux == 1) && (DC_Isolation_Sts_Aux == 1) && (HVBat_DC_CntctrStat_Aux == 1);
if handshake_ok
    HVBatChargeStat_Aux = 2; % charge allow current
end
% compute current state values used below
Vmax = max(voltageCell);
Temp_actual = max(temperatureCell);
SOC_actual = max(socCell);
if overTempFlag == true
    if Temp_actual <= (Ke_T_HighChrgTempThresholdIn - 5) && Temp_actual >= (Ke_T_LowChrgTempThresholdIn + 5)
        overTempFlag = false;
    end
end
% allow charging up to threshold + small hysteresis so we don't instantly cut
charge_allow_threshold = Ke_U_ChargeCompletionVlt;

% --- REFACTORED ---
% This block is now simpler and does not contain any fault detection.
if HVBatChargeStat_Aux == 2 && (ChargingSysSts == 1) && (Vmax < charge_allow_threshold) && chargeCompleteFlag == false && overTempFlag == false && Temp_actual < Ke_T_HighChrgTempThresholdIn && Temp_actual > Ke_T_LowChrgTempThresholdIn
    % Normal DC charging phase: populate MaxChargeCurrentAllowed (DC profile)
    MaxChargeCurrentAllowed = ParallelCellNum * interp2(SOC, Temp, DCChgCurrentProfile, SOC_actual, Temp_actual);
      
    MaxChargeCurrentAllowed_Aux = MaxChargeCurrentAllowed;
    HVBatChargeStat_Aux = 2; % charge allow current
    
% Charge completion    
elseif (ChargingSysSts == 1) && (Vmax >= (HVBatCell_Voltage_High_Thrsh - 0.01))
        % Enter CV-hold mode: provide PackCVCurrent to hold voltage steady
        MaxChargeCurrentAllowed_Aux = 0;
        HVBat_DC_CntctrStat_Aux = 0;   % Open
        HVBatChargeStat_Aux = 6;       % Complete
        DC_Isolation_Sts_Aux = 0;          % Enabled
        chargeCompleteFlag = true;

elseif Temp_actual >= Ke_T_HighChrgTempThresholdIn || Temp_actual <= Ke_T_LowChrgTempThresholdIn
    % Not in charging handshake/phase: ensure DCFC current 0
    MaxChargeCurrentAllowed_Aux = 0;
    overTempFlag = true;
    % chargeDisAllowFlag = true;
    % DC_Cntctr_Opn_Flag = true;
    HVBatChargeStat_Aux = 3;
    HVBat_DC_CntctrStat_Aux = 0;
end
%% ---------- Step: Charge completion ----------
% If BPCM DCFC completion criteria met (we model as per-cell threshold held),
% signal complete and set currents to 0.
% Improved completion logic with hysteresis
Vmax = max(voltageCell);
Vreset = Ke_U_ChargeCompletionVlt; 
if Vmax >= (HVBatCell_Voltage_High_Thrsh - 0.01)  % hysteresis
    if HVBatChargeTimer < 0
        HVBatChargeTimer = Time; % latch the first detection time
    end
    % Check if hold duration has passed
    if (Time - HVBatChargeTimer) >= Ke_t_ChargeCompletionHold
        MaxChargeCurrentAllowed_Aux = 0;
        HVBat_DC_CntctrStat_Aux = 0;   % Open
        HVBatChargeStat_Aux = 6;       % Complete
        DC_Isolation_Sts_Aux = 0;          % Enabled
        chargeCompleteFlag = true;
    end
elseif Vmax < Vreset
    % only reset the latch when voltage falls sufficiently below threshold (hysteresis)
    HVBatChargeTimer = -1;
end
%% ---------- Step: VCU commanded end of charging ----------
% If VCU indicates charge complete or stops commanding the DC contactor, BPCM opens and reports Open
if (ChargingSysSts == 3) || (PlugInChargingCmd == 0)
    if HVBatChargeTimer < 0
        HVBatChargeTimer = Time; % latch the first detection time
    end
    % Check if hold duration has passed
    if (Time - HVBatChargeTimer) >= Ke_t_ChargeCompletionHold
        MaxChargeCurrentAllowed_Aux = 0;
        HVBat_DC_CntctrStat_Aux = 0;   % Open
        HVBatChargeStat_Aux = 6;       % Complete
        DC_Isolation_Sts_Aux = 0;          % Enabled
        chargeCompleteFlag = true;
    end
elseif Vmax < Vreset
    % only reset the latch when voltage falls sufficiently below threshold (hysteresis)
    HVBatChargeTimer = -1;
end

%% ---------- Step: Global Fault Override (with request + open timers) ----------
% Fault condition that requires contactor open
fault_active = (Fault_General == 1) || (max(voltageCell) >= HVBatCell_Voltage_High_Thrsh);

Vmax = max(voltageCell); % ensure Vmax is defined here for clarity

if fault_active
    % 1) Immediately assert HVBatCntctrReq (request to return to normal or open)
    HVBatCntctrReq_Aux = 1;
    % latch start time for the request if not already latched
    if CntctrReqStartTime < 0
        CntctrReqStartTime = Time;
    end

    % 2) If request has persisted long enough, assert HVBatCntctrOpn notification
    if (Time - CntctrReqStartTime) >= Ke_t_CntctrReqDelay
        if HVBatCntctrOpn_Aux == 0
            % first time we enter the 'will-open' notification
            HVBatCntctrOpn_Aux = 1;
            CntctrOpnStartTime = Time;
        end
    end

    % 3) After the opn-notification delay, command coil open and force faulted status
    if HVBatCntctrOpn_Aux == 1 && (Time - CntctrOpnStartTime) >= Ke_t_DCFC_CntctrOpn
        DC_Cntctr_Opn_Aux = 1;       % Command the DC contactor coil open
        HVBat_DC_CntctrStat_Aux = 2; % Faulted (reported)
        HVBatChargeStat_Aux = 3;     % charge disallow
        DC_Isolation_Sts_Aux = 0;    % Isolation enabled
        MaxChargeCurrentAllowed_Aux = 0; % Disallow current
    end

else
    % no fault: clear all timers/flags and reset DC_Cntctr_Opn_Aux
    HVBatCntctrReq_Aux = 0;
    HVBatCntctrOpn_Aux = 0;
    CntctrReqStartTime = -1;
    CntctrOpnStartTime = -1;
    DC_Cntctr_Opn_Aux = 0;

    % If it was previously marked faulted/disallow, allow the state machine to re-evaluate
    if HVBat_DC_CntctrStat_Aux == 2
        HVBat_DC_CntctrStat_Aux = 0;
    end
    if HVBatChargeStat_Aux == 3
        HVBatChargeStat_Aux = 0;
    end
end

%% ---------- Assign outputs ----------
HVBat_DC_CntctrStat = HVBat_DC_CntctrStat_Aux;
HVBatChargeStat = HVBatChargeStat_Aux;
DC_Isolation_Sts = DC_Isolation_Sts_Aux;
MaxChargeCurrentAllowed = MaxChargeCurrentAllowed_Aux;
HVBat_DC_CntctrOpn = DC_Cntctr_Opn_Aux; 
HVBatCntctrOpn = HVBatCntctrOpn_Aux;   
HVBatCntctrReq = HVBatCntctrReq_Aux;   

% make sure no NaN
if isnan(MaxChargeCurrentAllowed), MaxChargeCurrentAllowed = 0; end
end

== matlab function Simulink_Program/Subsystem/Fault Indicators/MATLAB Function ==
  settings: updateMethod=DISCRETE
  data: K1 input; Fault_Contactor output; HVBatPack_DCFCVoltage input; HVBatPack_EDMVoltage input; PlugInChargingCmd input; MainHVBatCntctrCmd input; Fault_K1_Shorted_High output; Fault_K1_Shorted_Low output; Fault_K4_Shorted_High output; Fault_K4_Shorted_Low output; HVBatCntctrStat input
function [Fault_Contactor, Fault_K1_Shorted_High, Fault_K1_Shorted_Low, ...
          Fault_K4_Shorted_High, Fault_K4_Shorted_Low] = detectCntctrFaults( ...
    K1, HVBatPack_DCFCVoltage, HVBatPack_EDMVoltage, ...
    PlugInChargingCmd, MainHVBatCntctrCmd, HVBatCntctrStat)
%----------------------------------------------------
% Detect faults: K1 (Main) or K4 (DCFC) contactors
% Shorted High & Shorted Low conditions
%----------------------------------------------------
% -- Persistent Variables --
persistent last_Fault_K1_Shorted_High last_Fault_K1_Shorted_Low
persistent last_Fault_K4_Shorted_High last_Fault_K4_Shorted_Low
persistent K4_Shorted_Low_Counter
 
if isempty(last_Fault_K1_Shorted_High), last_Fault_K1_Shorted_High = 0; end
if isempty(last_Fault_K1_Shorted_Low),  last_Fault_K1_Shorted_Low  = 0; end
if isempty(last_Fault_K4_Shorted_High), last_Fault_K4_Shorted_High = 0; end
if isempty(last_Fault_K4_Shorted_Low),  last_Fault_K4_Shorted_Low  = 0; end
if isempty(K4_Shorted_Low_Counter),  K4_Shorted_Low_Counter  = 0; end

% -- Output Defaults --
Fault_Contactor = 0;
Fault_K1_Shorted_High = 0;  % 0 = OK, 1 = Shorted High
Fault_K1_Shorted_Low  = 0;  % 0 = OK, 1 = Shorted Low
Fault_K4_Shorted_High = 0;
Fault_K4_Shorted_Low  = 0;
 
% === Shorted High Fault Detection ===
% --- K1 Main Shorted High (after precharge, main OFF) ---
% if MainHVBatCntctrCmd == 0 && HVBatPack_EDMVoltage > 270
%     ShortedHighCounter = ShortedHighCounter + 1;
%     if ShortedHighCounter > 2
%         Fault_Contactor = 1;
%         Fault_K1_Shorted_High = 1;
%         last_Fault_K1_Shorted_High = 1;
%     end
% elseif MainHVBatCntctrCmd == 0
%     ShortedHighCounter = 0;
% end

if MainHVBatCntctrCmd == 0 && HVBatPack_EDMVoltage > 270
    % ShortedHighCounter = ShortedHighCounter + 1;
    % if ShortedHighCounter > 2
    Fault_K1_Shorted_High = 1;
    last_Fault_K1_Shorted_High = 1;
    % end
elseif MainHVBatCntctrCmd == 0 && HVBatPack_EDMVoltage <= 270
    Fault_K1_Shorted_High = 0;
    last_Fault_K1_Shorted_High = 0;
end
 
% --- K4 DCFC Shorted High (when not commanded, plug-in charging inactive) ---
if PlugInChargingCmd == 0 && K1 == 1 && HVBatPack_DCFCVoltage > 270
    Fault_K4_Shorted_High = 1;
    last_Fault_K4_Shorted_High = 1;
elseif PlugInChargingCmd == 0 && K1 == 1 && HVBatPack_DCFCVoltage <= 270
    Fault_K4_Shorted_High = 0;
    last_Fault_K4_Shorted_High = 0;
% elseif PlugInChargingCmd == 0 && K4 == 0 && HVBatPack_DCFCVoltage > 270
%     Fault_Contactor = 1;
%     Fault_K1_Shorted_High = 1;
%     last_Fault_K1_Shorted_High = 1;
end
 
% === Shorted Low Fault Detection ===
% --- K4 DCFC Shorted Low ---
if K1 == 1 && HVBatCntctrStat ~= 1 && PlugInChargingCmd == 1 && HVBatPack_DCFCVoltage <= 270
    if K4_Shorted_Low_Counter > 2
        Fault_Contactor = 1;
        Fault_K4_Shorted_Low = 1;
        last_Fault_K4_Shorted_Low = 1;
    elseif K4_Shorted_Low_Counter <= 2
        Fault_K4_Shorted_Low = 0;
        last_Fault_K4_Shorted_Low = 0;
    end
    K4_Shorted_Low_Counter = K4_Shorted_Low_Counter + 1;
end
 
% --- K1 Main Shorted Low ---
if HVBatCntctrStat ~= 1 && MainHVBatCntctrCmd == 1 && HVBatPack_EDMVoltage <= 270
    Fault_Contactor = 1;
    Fault_K1_Shorted_Low = 1;
    last_Fault_K1_Shorted_Low = 1;
end

if (MainHVBatCntctrCmd == 1 && HVBatPack_EDMVoltage > 270) || (MainHVBatCntctrCmd == 0 && HVBatPack_EDMVoltage <= 270)
    last_Fault_K1_Shorted_Low = 0;
    Fault_K1_Shorted_Low = 0; 
end

if (PlugInChargingCmd == 1 && HVBatPack_DCFCVoltage > 270) || (PlugInChargingCmd == 0 && HVBatPack_DCFCVoltage <= 270)
    last_Fault_K4_Shorted_Low = 0;
    Fault_K4_Shorted_Low = 0;
end

% === Latching Behaviour (Hold detected faults) ===
if last_Fault_K1_Shorted_High, Fault_K1_Shorted_High = 1; end
if last_Fault_K1_Shorted_Low,  Fault_K1_Shorted_Low  = 1; end
if last_Fault_K4_Shorted_High, Fault_K4_Shorted_High = 1; end
if last_Fault_K4_Shorted_Low,  Fault_K4_Shorted_Low  = 1; end
 
end
